simulink model "transmitter_lfsr256_v10"  (R2020a)
settings: Solver=VariableStepAuto  StartTime=0.0  StopTime=3000/128e6  RelTol=1e-3
PreLoadFcn:
  | setup_transmitter
InitFcn:
  | setup_transmitter
values from neighbouring files (symbols this model uses):
  tx_data = fi([1:1:1000], 0, 8, 0)    [setup_transmitter.m]
undefined symbols (not defined in the file or in its folder): a1, a2, a3, a4, a5, a6, a7, a8, AXI4, b1, b2, b3, b4, b5, b6, b7, b8, c1, c2, c3, c4, c5, c6, c7, c8, d1, d2, d3, d4, d5, d6, d7, d8, Lite
libraries: xbsIndex_r4 x30918, dspstat3 x66, hdlsllib x2, simulink x1
(part 4 of 157)

== system transmitter_lfsr256_v10/DUT/Algorithm/LFSR_512_3/LFSR256_imag/LFSR64_imag2/32LFSR_0 ==
  In1 : Inport
  8LFSR : Subsystem
  8LFSR2 : Subsystem
  8LFSR3 : Subsystem
  8LFSR4 : Subsystem
  AddSub : xbsIndex_r4/AddSub  mode=Addition  use_carryin=off  use_carryout=off  en=off  latency=as  precision=Full  arith_type=Unsigned  n_bits=16  bin_pt=14  quantization=Truncate  overflow=Wrap  use_behavioral_HDL=off  hw_selection=DSP48  pipelined=on  dbl_ovrd=off  block_type=addsub
  AddSub1 : xbsIndex_r4/AddSub  mode=Addition  use_carryin=off  use_carryout=off  en=off  latency=as  precision=Full  arith_type=Unsigned  n_bits=16  bin_pt=14  quantization=Truncate  overflow=Wrap  use_behavioral_HDL=off  hw_selection=DSP48  pipelined=on  dbl_ovrd=off  block_type=addsub
  AddSub2 : xbsIndex_r4/AddSub  mode=Addition  use_carryin=off  use_carryout=off  en=off  latency=as  precision=Full  arith_type=Unsigned  n_bits=16  bin_pt=14  quantization=Truncate  overflow=Wrap  use_behavioral_HDL=off  hw_selection=DSP48  pipelined=on  dbl_ovrd=off  block_type=addsub
  Out1 : Outport
  wires:
  8LFSR/1(Out1) -> AddSub/1
  8LFSR4/1(Out1) -> AddSub2/2
  8LFSR2/1(Out1) -> AddSub/2
  In1/1 -> 8LFSR3/1(In1), 8LFSR4/1(In1), 8LFSR/1(In1), 8LFSR2/1(In1)
  8LFSR3/1(Out1) -> AddSub2/1
  AddSub/1 -> AddSub1/1
  AddSub2/1 -> AddSub1/2
  AddSub1/1 -> Out1/1

== system transmitter_lfsr256_v10/DUT/Algorithm/LFSR_512_3/LFSR256_imag/LFSR64_imag2/32LFSR_1 ==
  In1 : Inport
  8LFSR : Subsystem
  8LFSR2 : Subsystem
  8LFSR3 : Subsystem
  8LFSR4 : Subsystem
  AddSub : xbsIndex_r4/AddSub  mode=Addition  use_carryin=off  use_carryout=off  en=off  latency=as  precision=Full  arith_type=Unsigned  n_bits=16  bin_pt=14  quantization=Truncate  overflow=Wrap  use_behavioral_HDL=off  hw_selection=DSP48  pipelined=on  dbl_ovrd=off  block_type=addsub
  AddSub1 : xbsIndex_r4/AddSub  mode=Addition  use_carryin=off  use_carryout=off  en=off  latency=as  precision=Full  arith_type=Unsigned  n_bits=16  bin_pt=14  quantization=Truncate  overflow=Wrap  use_behavioral_HDL=off  hw_selection=DSP48  pipelined=on  dbl_ovrd=off  block_type=addsub
  AddSub2 : xbsIndex_r4/AddSub  mode=Addition  use_carryin=off  use_carryout=off  en=off  latency=as  precision=Full  arith_type=Unsigned  n_bits=16  bin_pt=14  quantization=Truncate  overflow=Wrap  use_behavioral_HDL=off  hw_selection=DSP48  pipelined=on  dbl_ovrd=off  block_type=addsub
  Out1 : Outport
  wires:
  8LFSR/1(Out1) -> AddSub/1
  8LFSR4/1(Out1) -> AddSub2/2
  8LFSR2/1(Out1) -> AddSub/2
  In1/1 -> 8LFSR3/1(In1), 8LFSR4/1(In1), 8LFSR/1(In1), 8LFSR2/1(In1)
  8LFSR3/1(Out1) -> AddSub2/1
  AddSub/1 -> AddSub1/1
  AddSub2/1 -> AddSub1/2
  AddSub1/1 -> Out1/1

== system transmitter_lfsr256_v10/DUT/Algorithm/LFSR_512_3/LFSR256_imag/LFSR64_imag3/32LFSR_0 ==
  In1 : Inport
  8LFSR : Subsystem
  8LFSR2 : Subsystem
  8LFSR3 : Subsystem
  8LFSR4 : Subsystem
  AddSub : xbsIndex_r4/AddSub  mode=Addition  use_carryin=off  use_carryout=off  en=off  latency=as  precision=Full  arith_type=Unsigned  n_bits=16  bin_pt=14  quantization=Truncate  overflow=Wrap  use_behavioral_HDL=off  hw_selection=DSP48  pipelined=on  dbl_ovrd=off  block_type=addsub
  AddSub1 : xbsIndex_r4/AddSub  mode=Addition  use_carryin=off  use_carryout=off  en=off  latency=as  precision=Full  arith_type=Unsigned  n_bits=16  bin_pt=14  quantization=Truncate  overflow=Wrap  use_behavioral_HDL=off  hw_selection=DSP48  pipelined=on  dbl_ovrd=off  block_type=addsub
  AddSub2 : xbsIndex_r4/AddSub  mode=Addition  use_carryin=off  use_carryout=off  en=off  latency=as  precision=Full  arith_type=Unsigned  n_bits=16  bin_pt=14  quantization=Truncate  overflow=Wrap  use_behavioral_HDL=off  hw_selection=DSP48  pipelined=on  dbl_ovrd=off  block_type=addsub
  Out1 : Outport
  wires:
  8LFSR/1(Out1) -> AddSub/1
  8LFSR4/1(Out1) -> AddSub2/2
  8LFSR2/1(Out1) -> AddSub/2
  In1/1 -> 8LFSR3/1(In1), 8LFSR4/1(In1), 8LFSR/1(In1), 8LFSR2/1(In1)
  8LFSR3/1(Out1) -> AddSub2/1
  AddSub/1 -> AddSub1/1
  AddSub2/1 -> AddSub1/2
  AddSub1/1 -> Out1/1

== system transmitter_lfsr256_v10/DUT/Algorithm/LFSR_512_3/LFSR256_imag/LFSR64_imag3/32LFSR_1 ==
  In1 : Inport
  8LFSR : Subsystem
  8LFSR2 : Subsystem
  8LFSR3 : Subsystem
  8LFSR4 : Subsystem
  AddSub : xbsIndex_r4/AddSub  mode=Addition  use_carryin=off  use_carryout=off  en=off  latency=as  precision=Full  arith_type=Unsigned  n_bits=16  bin_pt=14  quantization=Truncate  overflow=Wrap  use_behavioral_HDL=off  hw_selection=DSP48  pipelined=on  dbl_ovrd=off  block_type=addsub
  AddSub1 : xbsIndex_r4/AddSub  mode=Addition  use_carryin=off  use_carryout=off  en=off  latency=as  precision=Full  arith_type=Unsigned  n_bits=16  bin_pt=14  quantization=Truncate  overflow=Wrap  use_behavioral_HDL=off  hw_selection=DSP48  pipelined=on  dbl_ovrd=off  block_type=addsub
  AddSub2 : xbsIndex_r4/AddSub  mode=Addition  use_carryin=off  use_carryout=off  en=off  latency=as  precision=Full  arith_type=Unsigned  n_bits=16  bin_pt=14  quantization=Truncate  overflow=Wrap  use_behavioral_HDL=off  hw_selection=DSP48  pipelined=on  dbl_ovrd=off  block_type=addsub
  Out1 : Outport
  wires:
  8LFSR/1(Out1) -> AddSub/1
  8LFSR4/1(Out1) -> AddSub2/2
  8LFSR2/1(Out1) -> AddSub/2
  In1/1 -> 8LFSR3/1(In1), 8LFSR4/1(In1), 8LFSR/1(In1), 8LFSR2/1(In1)
  8LFSR3/1(Out1) -> AddSub2/1
  AddSub/1 -> AddSub1/1
  AddSub2/1 -> AddSub1/2
  AddSub1/1 -> Out1/1

== system transmitter_lfsr256_v10/DUT/Algorithm/LFSR_512_3/LFSR256_imag/LFSR64_imag4/32LFSR_0 ==
  In1 : Inport
  8LFSR : Subsystem
  8LFSR2 : Subsystem
  8LFSR3 : Subsystem
  8LFSR4 : Subsystem
  AddSub : xbsIndex_r4/AddSub  mode=Addition  use_carryin=off  use_carryout=off  en=off  latency=as  precision=Full  arith_type=Unsigned  n_bits=16  bin_pt=14  quantization=Truncate  overflow=Wrap  use_behavioral_HDL=off  hw_selection=DSP48  pipelined=on  dbl_ovrd=off  block_type=addsub
  AddSub1 : xbsIndex_r4/AddSub  mode=Addition  use_carryin=off  use_carryout=off  en=off  latency=as  precision=Full  arith_type=Unsigned  n_bits=16  bin_pt=14  quantization=Truncate  overflow=Wrap  use_behavioral_HDL=off  hw_selection=DSP48  pipelined=on  dbl_ovrd=off  block_type=addsub
  AddSub2 : xbsIndex_r4/AddSub  mode=Addition  use_carryin=off  use_carryout=off  en=off  latency=as  precision=Full  arith_type=Unsigned  n_bits=16  bin_pt=14  quantization=Truncate  overflow=Wrap  use_behavioral_HDL=off  hw_selection=DSP48  pipelined=on  dbl_ovrd=off  block_type=addsub
  Out1 : Outport
  wires:
  8LFSR/1(Out1) -> AddSub/1
  8LFSR4/1(Out1) -> AddSub2/2
  8LFSR2/1(Out1) -> AddSub/2
  In1/1 -> 8LFSR3/1(In1), 8LFSR4/1(In1), 8LFSR/1(In1), 8LFSR2/1(In1)
  8LFSR3/1(Out1) -> AddSub2/1
  AddSub/1 -> AddSub1/1
  AddSub2/1 -> AddSub1/2
  AddSub1/1 -> Out1/1

== system transmitter_lfsr256_v10/DUT/Algorithm/LFSR_512_3/LFSR256_imag/LFSR64_imag4/32LFSR_1 ==
  In1 : Inport
  8LFSR : Subsystem
  8LFSR2 : Subsystem
  8LFSR3 : Subsystem
  8LFSR4 : Subsystem
  AddSub : xbsIndex_r4/AddSub  mode=Addition  use_carryin=off  use_carryout=off  en=off  latency=as  precision=Full  arith_type=Unsigned  n_bits=16  bin_pt=14  quantization=Truncate  overflow=Wrap  use_behavioral_HDL=off  hw_selection=DSP48  pipelined=on  dbl_ovrd=off  block_type=addsub
  AddSub1 : xbsIndex_r4/AddSub  mode=Addition  use_carryin=off  use_carryout=off  en=off  latency=as  precision=Full  arith_type=Unsigned  n_bits=16  bin_pt=14  quantization=Truncate  overflow=Wrap  use_behavioral_HDL=off  hw_selection=DSP48  pipelined=on  dbl_ovrd=off  block_type=addsub
  AddSub2 : xbsIndex_r4/AddSub  mode=Addition  use_carryin=off  use_carryout=off  en=off  latency=as  precision=Full  arith_type=Unsigned  n_bits=16  bin_pt=14  quantization=Truncate  overflow=Wrap  use_behavioral_HDL=off  hw_selection=DSP48  pipelined=on  dbl_ovrd=off  block_type=addsub
  Out1 : Outport
  wires:
  8LFSR/1(Out1) -> AddSub/1
  8LFSR4/1(Out1) -> AddSub2/2
  8LFSR2/1(Out1) -> AddSub/2
  In1/1 -> 8LFSR3/1(In1), 8LFSR4/1(In1), 8LFSR/1(In1), 8LFSR2/1(In1)
  8LFSR3/1(Out1) -> AddSub2/1
  AddSub/1 -> AddSub1/1
  AddSub2/1 -> AddSub1/2
  AddSub1/1 -> Out1/1

== system transmitter_lfsr256_v10/DUT/Algorithm/LFSR_512_3/LFSR256_real/LFSR64_imag1/32LFSR_0 ==
  In1 : Inport
  8LFSR : Subsystem
  8LFSR2 : Subsystem
  8LFSR3 : Subsystem
  8LFSR4 : Subsystem
  AddSub : xbsIndex_r4/AddSub  mode=Addition  use_carryin=off  use_carryout=off  en=off  latency=as  precision=Full  arith_type=Unsigned  n_bits=16  bin_pt=14  quantization=Truncate  overflow=Wrap  use_behavioral_HDL=off  hw_selection=DSP48  pipelined=on  dbl_ovrd=off  block_type=addsub
  AddSub1 : xbsIndex_r4/AddSub  mode=Addition  use_carryin=off  use_carryout=off  en=off  latency=as  precision=Full  arith_type=Unsigned  n_bits=16  bin_pt=14  quantization=Truncate  overflow=Wrap  use_behavioral_HDL=off  hw_selection=DSP48  pipelined=on  dbl_ovrd=off  block_type=addsub
  AddSub2 : xbsIndex_r4/AddSub  mode=Addition  use_carryin=off  use_carryout=off  en=off  latency=as  precision=Full  arith_type=Unsigned  n_bits=16  bin_pt=14  quantization=Truncate  overflow=Wrap  use_behavioral_HDL=off  hw_selection=DSP48  pipelined=on  dbl_ovrd=off  block_type=addsub
  Out1 : Outport
  wires:
  8LFSR/1(Out1) -> AddSub/1
  8LFSR4/1(Out1) -> AddSub2/2
  8LFSR2/1(Out1) -> AddSub/2
  In1/1 -> 8LFSR3/1(In1), 8LFSR4/1(In1), 8LFSR/1(In1), 8LFSR2/1(In1)
  8LFSR3/1(Out1) -> AddSub2/1
  AddSub/1 -> AddSub1/1
  AddSub2/1 -> AddSub1/2
  AddSub1/1 -> Out1/1

== system transmitter_lfsr256_v10/DUT/Algorithm/LFSR_512_3/LFSR256_real/LFSR64_imag1/32LFSR_1 ==
  In1 : Inport
  8LFSR : Subsystem
  8LFSR2 : Subsystem
  8LFSR3 : Subsystem
  8LFSR4 : Subsystem
  AddSub : xbsIndex_r4/AddSub  mode=Addition  use_carryin=off  use_carryout=off  en=off  latency=as  precision=Full  arith_type=Unsigned  n_bits=16  bin_pt=14  quantization=Truncate  overflow=Wrap  use_behavioral_HDL=off  hw_selection=DSP48  pipelined=on  dbl_ovrd=off  block_type=addsub
  AddSub1 : xbsIndex_r4/AddSub  mode=Addition  use_carryin=off  use_carryout=off  en=off  latency=as  precision=Full  arith_type=Unsigned  n_bits=16  bin_pt=14  quantization=Truncate  overflow=Wrap  use_behavioral_HDL=off  hw_selection=DSP48  pipelined=on  dbl_ovrd=off  block_type=addsub
  AddSub2 : xbsIndex_r4/AddSub  mode=Addition  use_carryin=off  use_carryout=off  en=off  latency=as  precision=Full  arith_type=Unsigned  n_bits=16  bin_pt=14  quantization=Truncate  overflow=Wrap  use_behavioral_HDL=off  hw_selection=DSP48  pipelined=on  dbl_ovrd=off  block_type=addsub
  Out1 : Outport
  wires:
  8LFSR/1(Out1) -> AddSub/1
  8LFSR4/1(Out1) -> AddSub2/2
  8LFSR2/1(Out1) -> AddSub/2
  In1/1 -> 8LFSR3/1(In1), 8LFSR4/1(In1), 8LFSR/1(In1), 8LFSR2/1(In1)
  8LFSR3/1(Out1) -> AddSub2/1
  AddSub/1 -> AddSub1/1
  AddSub2/1 -> AddSub1/2
  AddSub1/1 -> Out1/1

== system transmitter_lfsr256_v10/DUT/Algorithm/LFSR_512_3/LFSR256_real/LFSR64_imag2/32LFSR_0 ==
  In1 : Inport
  8LFSR : Subsystem
  8LFSR2 : Subsystem
  8LFSR3 : Subsystem
  8LFSR4 : Subsystem
  AddSub : xbsIndex_r4/AddSub  mode=Addition  use_carryin=off  use_carryout=off  en=off  latency=as  precision=Full  arith_type=Unsigned  n_bits=16  bin_pt=14  quantization=Truncate  overflow=Wrap  use_behavioral_HDL=off  hw_selection=DSP48  pipelined=on  dbl_ovrd=off  block_type=addsub
  AddSub1 : xbsIndex_r4/AddSub  mode=Addition  use_carryin=off  use_carryout=off  en=off  latency=as  precision=Full  arith_type=Unsigned  n_bits=16  bin_pt=14  quantization=Truncate  overflow=Wrap  use_behavioral_HDL=off  hw_selection=DSP48  pipelined=on  dbl_ovrd=off  block_type=addsub
  AddSub2 : xbsIndex_r4/AddSub  mode=Addition  use_carryin=off  use_carryout=off  en=off  latency=as  precision=Full  arith_type=Unsigned  n_bits=16  bin_pt=14  quantization=Truncate  overflow=Wrap  use_behavioral_HDL=off  hw_selection=DSP48  pipelined=on  dbl_ovrd=off  block_type=addsub
  Out1 : Outport
  wires:
  8LFSR/1(Out1) -> AddSub/1
  8LFSR4/1(Out1) -> AddSub2/2
  8LFSR2/1(Out1) -> AddSub/2
  In1/1 -> 8LFSR3/1(In1), 8LFSR4/1(In1), 8LFSR/1(In1), 8LFSR2/1(In1)
  8LFSR3/1(Out1) -> AddSub2/1
  AddSub/1 -> AddSub1/1
  AddSub2/1 -> AddSub1/2
  AddSub1/1 -> Out1/1

== system transmitter_lfsr256_v10/DUT/Algorithm/LFSR_512_3/LFSR256_real/LFSR64_imag2/32LFSR_1 ==
  In1 : Inport
  8LFSR : Subsystem
  8LFSR2 : Subsystem
  8LFSR3 : Subsystem
  8LFSR4 : Subsystem
  AddSub : xbsIndex_r4/AddSub  mode=Addition  use_carryin=off  use_carryout=off  en=off  latency=as  precision=Full  arith_type=Unsigned  n_bits=16  bin_pt=14  quantization=Truncate  overflow=Wrap  use_behavioral_HDL=off  hw_selection=DSP48  pipelined=on  dbl_ovrd=off  block_type=addsub
  AddSub1 : xbsIndex_r4/AddSub  mode=Addition  use_carryin=off  use_carryout=off  en=off  latency=as  precision=Full  arith_type=Unsigned  n_bits=16  bin_pt=14  quantization=Truncate  overflow=Wrap  use_behavioral_HDL=off  hw_selection=DSP48  pipelined=on  dbl_ovrd=off  block_type=addsub
  AddSub2 : xbsIndex_r4/AddSub  mode=Addition  use_carryin=off  use_carryout=off  en=off  latency=as  precision=Full  arith_type=Unsigned  n_bits=16  bin_pt=14  quantization=Truncate  overflow=Wrap  use_behavioral_HDL=off  hw_selection=DSP48  pipelined=on  dbl_ovrd=off  block_type=addsub
  Out1 : Outport
  wires:
  8LFSR/1(Out1) -> AddSub/1
  8LFSR4/1(Out1) -> AddSub2/2
  8LFSR2/1(Out1) -> AddSub/2
  In1/1 -> 8LFSR3/1(In1), 8LFSR4/1(In1), 8LFSR/1(In1), 8LFSR2/1(In1)
  8LFSR3/1(Out1) -> AddSub2/1
  AddSub/1 -> AddSub1/1
  AddSub2/1 -> AddSub1/2
  AddSub1/1 -> Out1/1

== system transmitter_lfsr256_v10/DUT/Algorithm/LFSR_512_3/LFSR256_real/LFSR64_imag3/32LFSR_0 ==
  In1 : Inport
  8LFSR : Subsystem
  8LFSR2 : Subsystem
  8LFSR3 : Subsystem
  8LFSR4 : Subsystem
  AddSub : xbsIndex_r4/AddSub  mode=Addition  use_carryin=off  use_carryout=off  en=off  latency=as  precision=Full  arith_type=Unsigned  n_bits=16  bin_pt=14  quantization=Truncate  overflow=Wrap  use_behavioral_HDL=off  hw_selection=DSP48  pipelined=on  dbl_ovrd=off  block_type=addsub
  AddSub1 : xbsIndex_r4/AddSub  mode=Addition  use_carryin=off  use_carryout=off  en=off  latency=as  precision=Full  arith_type=Unsigned  n_bits=16  bin_pt=14  quantization=Truncate  overflow=Wrap  use_behavioral_HDL=off  hw_selection=DSP48  pipelined=on  dbl_ovrd=off  block_type=addsub
  AddSub2 : xbsIndex_r4/AddSub  mode=Addition  use_carryin=off  use_carryout=off  en=off  latency=as  precision=Full  arith_type=Unsigned  n_bits=16  bin_pt=14  quantization=Truncate  overflow=Wrap  use_behavioral_HDL=off  hw_selection=DSP48  pipelined=on  dbl_ovrd=off  block_type=addsub
  Out1 : Outport
  wires:
  8LFSR/1(Out1) -> AddSub/1
  8LFSR4/1(Out1) -> AddSub2/2
  8LFSR2/1(Out1) -> AddSub/2
  In1/1 -> 8LFSR3/1(In1), 8LFSR4/1(In1), 8LFSR/1(In1), 8LFSR2/1(In1)
  8LFSR3/1(Out1) -> AddSub2/1
  AddSub/1 -> AddSub1/1
  AddSub2/1 -> AddSub1/2
  AddSub1/1 -> Out1/1

== system transmitter_lfsr256_v10/DUT/Algorithm/LFSR_512_3/LFSR256_real/LFSR64_imag3/32LFSR_1 ==
  In1 : Inport
  8LFSR : Subsystem
  8LFSR2 : Subsystem
  8LFSR3 : Subsystem
  8LFSR4 : Subsystem
  AddSub : xbsIndex_r4/AddSub  mode=Addition  use_carryin=off  use_carryout=off  en=off  latency=as  precision=Full  arith_type=Unsigned  n_bits=16  bin_pt=14  quantization=Truncate  overflow=Wrap  use_behavioral_HDL=off  hw_selection=DSP48  pipelined=on  dbl_ovrd=off  block_type=addsub
  AddSub1 : xbsIndex_r4/AddSub  mode=Addition  use_carryin=off  use_carryout=off  en=off  latency=as  precision=Full  arith_type=Unsigned  n_bits=16  bin_pt=14  quantization=Truncate  overflow=Wrap  use_behavioral_HDL=off  hw_selection=DSP48  pipelined=on  dbl_ovrd=off  block_type=addsub
  AddSub2 : xbsIndex_r4/AddSub  mode=Addition  use_carryin=off  use_carryout=off  en=off  latency=as  precision=Full  arith_type=Unsigned  n_bits=16  bin_pt=14  quantization=Truncate  overflow=Wrap  use_behavioral_HDL=off  hw_selection=DSP48  pipelined=on  dbl_ovrd=off  block_type=addsub
  Out1 : Outport
  wires:
  8LFSR/1(Out1) -> AddSub/1
  8LFSR4/1(Out1) -> AddSub2/2
  8LFSR2/1(Out1) -> AddSub/2
  In1/1 -> 8LFSR3/1(In1), 8LFSR4/1(In1), 8LFSR/1(In1), 8LFSR2/1(In1)
  8LFSR3/1(Out1) -> AddSub2/1
  AddSub/1 -> AddSub1/1
  AddSub2/1 -> AddSub1/2
  AddSub1/1 -> Out1/1

== system transmitter_lfsr256_v10/DUT/Algorithm/LFSR_512_3/LFSR256_real/LFSR64_imag4/32LFSR_0 ==
  In1 : Inport
  8LFSR : Subsystem
  8LFSR2 : Subsystem
  8LFSR3 : Subsystem
  8LFSR4 : Subsystem
  AddSub : xbsIndex_r4/AddSub  mode=Addition  use_carryin=off  use_carryout=off  en=off  latency=as  precision=Full  arith_type=Unsigned  n_bits=16  bin_pt=14  quantization=Truncate  overflow=Wrap  use_behavioral_HDL=off  hw_selection=DSP48  pipelined=on  dbl_ovrd=off  block_type=addsub
  AddSub1 : xbsIndex_r4/AddSub  mode=Addition  use_carryin=off  use_carryout=off  en=off  latency=as  precision=Full  arith_type=Unsigned  n_bits=16  bin_pt=14  quantization=Truncate  overflow=Wrap  use_behavioral_HDL=off  hw_selection=DSP48  pipelined=on  dbl_ovrd=off  block_type=addsub
  AddSub2 : xbsIndex_r4/AddSub  mode=Addition  use_carryin=off  use_carryout=off  en=off  latency=as  precision=Full  arith_type=Unsigned  n_bits=16  bin_pt=14  quantization=Truncate  overflow=Wrap  use_behavioral_HDL=off  hw_selection=DSP48  pipelined=on  dbl_ovrd=off  block_type=addsub
  Out1 : Outport
  wires:
  8LFSR/1(Out1) -> AddSub/1
  8LFSR4/1(Out1) -> AddSub2/2
  8LFSR2/1(Out1) -> AddSub/2
  In1/1 -> 8LFSR3/1(In1), 8LFSR4/1(In1), 8LFSR/1(In1), 8LFSR2/1(In1)
  8LFSR3/1(Out1) -> AddSub2/1
  AddSub/1 -> AddSub1/1
  AddSub2/1 -> AddSub1/2
  AddSub1/1 -> Out1/1

== system transmitter_lfsr256_v10/DUT/Algorithm/LFSR_512_3/LFSR256_real/LFSR64_imag4/32LFSR_1 ==
  In1 : Inport
  8LFSR : Subsystem
  8LFSR2 : Subsystem
  8LFSR3 : Subsystem
  8LFSR4 : Subsystem
  AddSub : xbsIndex_r4/AddSub  mode=Addition  use_carryin=off  use_carryout=off  en=off  latency=as  precision=Full  arith_type=Unsigned  n_bits=16  bin_pt=14  quantization=Truncate  overflow=Wrap  use_behavioral_HDL=off  hw_selection=DSP48  pipelined=on  dbl_ovrd=off  block_type=addsub
  AddSub1 : xbsIndex_r4/AddSub  mode=Addition  use_carryin=off  use_carryout=off  en=off  latency=as  precision=Full  arith_type=Unsigned  n_bits=16  bin_pt=14  quantization=Truncate  overflow=Wrap  use_behavioral_HDL=off  hw_selection=DSP48  pipelined=on  dbl_ovrd=off  block_type=addsub
  AddSub2 : xbsIndex_r4/AddSub  mode=Addition  use_carryin=off  use_carryout=off  en=off  latency=as  precision=Full  arith_type=Unsigned  n_bits=16  bin_pt=14  quantization=Truncate  overflow=Wrap  use_behavioral_HDL=off  hw_selection=DSP48  pipelined=on  dbl_ovrd=off  block_type=addsub
  Out1 : Outport
  wires:
  8LFSR/1(Out1) -> AddSub/1
  8LFSR4/1(Out1) -> AddSub2/2
  8LFSR2/1(Out1) -> AddSub/2
  In1/1 -> 8LFSR3/1(In1), 8LFSR4/1(In1), 8LFSR/1(In1), 8LFSR2/1(In1)
  8LFSR3/1(Out1) -> AddSub2/1
  AddSub/1 -> AddSub1/1
  AddSub2/1 -> AddSub1/2
  AddSub1/1 -> Out1/1

== system transmitter_lfsr256_v10/DUT/Algorithm/LFSR_512_0/LFSR256_imag/LFSR64_imag1/32LFSR_0/8LFSR ==
  In1 : Inport
  AddSub{,1..6} (7 alike) : xbsIndex_r4/AddSub  mode=Addition  use_carryin=off  use_carryout=off  en=off  latency=as  precision=Full  arith_type=Unsigned  n_bits=16  bin_pt=14  quantization=Truncate  overflow=Wrap  use_behavioral_HDL=off  hw_selection=DSP48  pipelined=on  dbl_ovrd=off  block_type=addsub
  LFSR_SIMPLE : Subsystem
  LFSR_SIMPLE1 : Subsystem
  LFSR_SIMPLE2 : Subsystem
  LFSR_SIMPLE3 : Subsystem
  LFSR_SIMPLE4 : Subsystem
  LFSR_SIMPLE5 : Subsystem
  LFSR_SIMPLE6 : Subsystem
  LFSR_SIMPLE7 : Subsystem
  Out1 : Outport
  wires:
  LFSR_SIMPLE3/1(Out1) -> AddSub2/2
  LFSR_SIMPLE5/1(Out1) -> AddSub4/2
  LFSR_SIMPLE1/1(Out1) -> AddSub/2
  LFSR_SIMPLE/1(Out1) -> AddSub/1
  AddSub6/1 -> Out1/1
  In1/1 -> LFSR_SIMPLE7/1(In1), LFSR_SIMPLE6/1(In1), LFSR_SIMPLE5/1(In1), LFSR_SIMPLE4/1(In1), LFSR_SIMPLE3/1(In1), LFSR_SIMPLE2/1(In1), LFSR_SIMPLE1/1(In1), LFSR_SIMPLE/1(In1)
  LFSR_SIMPLE2/1(Out1) -> AddSub2/1
  LFSR_SIMPLE4/1(Out1) -> AddSub4/1
  LFSR_SIMPLE7/1(Out1) -> AddSub5/2
  LFSR_SIMPLE6/1(Out1) -> AddSub5/1
  AddSub/1 -> AddSub1/1
  AddSub2/1 -> AddSub1/2
  AddSub4/1 -> AddSub3/1
  AddSub5/1 -> AddSub3/2
  AddSub1/1 -> AddSub6/1
  AddSub3/1 -> AddSub6/2

== system transmitter_lfsr256_v10/DUT/Algorithm/LFSR_512_0/LFSR256_imag/LFSR64_imag1/32LFSR_0/8LFSR2 ==
  In1 : Inport
  AddSub{,1..6} (7 alike) : xbsIndex_r4/AddSub  mode=Addition  use_carryin=off  use_carryout=off  en=off  latency=as  precision=Full  arith_type=Unsigned  n_bits=16  bin_pt=14  quantization=Truncate  overflow=Wrap  use_behavioral_HDL=off  hw_selection=DSP48  pipelined=on  dbl_ovrd=off  block_type=addsub
  LFSR_SIMPLE : Subsystem
  LFSR_SIMPLE1 : Subsystem
  LFSR_SIMPLE2 : Subsystem
  LFSR_SIMPLE3 : Subsystem
  LFSR_SIMPLE4 : Subsystem
  LFSR_SIMPLE5 : Subsystem
  LFSR_SIMPLE6 : Subsystem
  LFSR_SIMPLE7 : Subsystem
  Out1 : Outport
  wires:
  LFSR_SIMPLE3/1(Out1) -> AddSub2/2
  AddSub/1 -> AddSub1/1
  AddSub4/1 -> AddSub5/1
  LFSR_SIMPLE5/1(Out1) -> AddSub4/2
  LFSR_SIMPLE7/1(Out1) -> AddSub6/2
  LFSR_SIMPLE1/1(Out1) -> AddSub/2
  LFSR_SIMPLE/1(Out1) -> AddSub/1
  In1/1 -> LFSR_SIMPLE4/1(In1), LFSR_SIMPLE5/1(In1), LFSR_SIMPLE6/1(In1), LFSR_SIMPLE7/1(In1), LFSR_SIMPLE2/1(In1), LFSR_SIMPLE1/1(In1), LFSR_SIMPLE/1(In1), LFSR_SIMPLE3/1(In1)
  LFSR_SIMPLE2/1(Out1) -> AddSub2/1
  AddSub2/1 -> AddSub1/2
  LFSR_SIMPLE4/1(Out1) -> AddSub4/1
  LFSR_SIMPLE6/1(Out1) -> AddSub6/1
  AddSub6/1 -> AddSub5/2
  AddSub1/1 -> AddSub3/1
  AddSub5/1 -> AddSub3/2
  AddSub3/1 -> Out1/1

== system transmitter_lfsr256_v10/DUT/Algorithm/LFSR_512_0/LFSR256_imag/LFSR64_imag1/32LFSR_0/8LFSR3 ==
  In1 : Inport
  AddSub{,1..6} (7 alike) : xbsIndex_r4/AddSub  mode=Addition  use_carryin=off  use_carryout=off  en=off  latency=as  precision=Full  arith_type=Unsigned  n_bits=16  bin_pt=14  quantization=Truncate  overflow=Wrap  use_behavioral_HDL=off  hw_selection=DSP48  pipelined=on  dbl_ovrd=off  block_type=addsub
  LFSR_SIMPLE : Subsystem
  LFSR_SIMPLE1 : Subsystem
  LFSR_SIMPLE2 : Subsystem
  LFSR_SIMPLE3 : Subsystem
  LFSR_SIMPLE4 : Subsystem
  LFSR_SIMPLE5 : Subsystem
  LFSR_SIMPLE6 : Subsystem
  LFSR_SIMPLE7 : Subsystem
  Out1 : Outport
  wires:
  LFSR_SIMPLE3/1(Out1) -> AddSub2/2
  AddSub/1 -> AddSub1/1
  AddSub4/1 -> AddSub5/1
  LFSR_SIMPLE5/1(Out1) -> AddSub4/2
  LFSR_SIMPLE7/1(Out1) -> AddSub6/2
  LFSR_SIMPLE1/1(Out1) -> AddSub/2
  LFSR_SIMPLE/1(Out1) -> AddSub/1
  In1/1 -> LFSR_SIMPLE4/1(In1), LFSR_SIMPLE5/1(In1), LFSR_SIMPLE6/1(In1), LFSR_SIMPLE7/1(In1), LFSR_SIMPLE2/1(In1), LFSR_SIMPLE1/1(In1), LFSR_SIMPLE/1(In1), LFSR_SIMPLE3/1(In1)
  LFSR_SIMPLE2/1(Out1) -> AddSub2/1
  AddSub2/1 -> AddSub1/2
  LFSR_SIMPLE4/1(Out1) -> AddSub4/1
  LFSR_SIMPLE6/1(Out1) -> AddSub6/1
  AddSub6/1 -> AddSub5/2
  AddSub5/1 -> AddSub3/2
  AddSub1/1 -> AddSub3/1
  AddSub3/1 -> Out1/1

== system transmitter_lfsr256_v10/DUT/Algorithm/LFSR_512_0/LFSR256_imag/LFSR64_imag1/32LFSR_0/8LFSR4 ==
  In1 : Inport
  AddSub{,1..6} (7 alike) : xbsIndex_r4/AddSub  mode=Addition  use_carryin=off  use_carryout=off  en=off  latency=as  precision=Full  arith_type=Unsigned  n_bits=16  bin_pt=14  quantization=Truncate  overflow=Wrap  use_behavioral_HDL=off  hw_selection=DSP48  pipelined=on  dbl_ovrd=off  block_type=addsub
  LFSR_SIMPLE : Subsystem
  LFSR_SIMPLE1 : Subsystem
  LFSR_SIMPLE2 : Subsystem
  LFSR_SIMPLE3 : Subsystem
  LFSR_SIMPLE4 : Subsystem
  LFSR_SIMPLE5 : Subsystem
  LFSR_SIMPLE6 : Subsystem
  LFSR_SIMPLE7 : Subsystem
  Out1 : Outport
  wires:
  LFSR_SIMPLE3/1(Out1) -> AddSub2/2
  AddSub/1 -> AddSub1/1
  AddSub4/1 -> AddSub5/1
  LFSR_SIMPLE5/1(Out1) -> AddSub4/2
  LFSR_SIMPLE7/1(Out1) -> AddSub6/2
  LFSR_SIMPLE1/1(Out1) -> AddSub/2
  LFSR_SIMPLE/1(Out1) -> AddSub/1
  In1/1 -> LFSR_SIMPLE4/1(In1), LFSR_SIMPLE6/1(In1), LFSR_SIMPLE7/1(In1), LFSR_SIMPLE5/1(In1), LFSR_SIMPLE2/1(In1), LFSR_SIMPLE1/1(In1), LFSR_SIMPLE/1(In1), LFSR_SIMPLE3/1(In1)
  LFSR_SIMPLE2/1(Out1) -> AddSub2/1
  AddSub2/1 -> AddSub1/2
  LFSR_SIMPLE4/1(Out1) -> AddSub4/1
  LFSR_SIMPLE6/1(Out1) -> AddSub6/1
  AddSub6/1 -> AddSub5/2
  AddSub1/1 -> AddSub3/1
  AddSub5/1 -> AddSub3/2
  AddSub3/1 -> Out1/1
